simulink model "sma_corr"  (Simulink 8.0)
settings: Solver=VariableStepDiscrete  SolverMode=SingleTasking  StartTime=0.0  StopTime=1000  RelTol=1e-3
PreLoadFcn:
  | % Set this to 1 if simulating
  | simulating = 0;
  | 
  | % Overall parameters
  | n_comp = 128;
  | 
  | % Simulation
  | if (simulating)
  |     n_tvg_frames = 4;
  |     frames_per_vector = 4*8;
  |     vectors_per_wstep = 2;
  |     total_wsteps = 1; % 2^n
  |     % Laura's params
  |     x_ctr = 12;
  |     x_data_ctr = x_ctr-5;
  |     rx_addr_ctr = x_data_ctr+2;
  |     tx_addr_ctr = x_data_ctr+2;
  | else
  |     n_tvg_frames = 256;
  |     frames_per_vector = 256*8;
  |     vectors_per_wstep = 11;
  |     total_wsteps = 6; % 2^n
  |     % Laura's params
  |     x_ctr = 32;
  |     x_data_ctr = 18;
  |     rx_addr_ctr = x_data_ctr+2;
  |     tx_addr_ctr = x_data_ctr+2;
  | end
  | 
  | sb_states = repmat([ones(1, 256) zeros(1, 256)], 1, 2^(total_wsteps-1));
InitFcn:
  | % Get the XSG object to find the clock source
  | xps_xsg_blks = find_system(gcs,'FollowLinks','on','LookUnderMasks','all', 'SearchDepth',1, 'Tag','xps:xsg');
  | xsg_obj = xps_block(xps_xsg_blks{1}, {});
  | xsg_obj = xps_xsg(xsg_obj);
  | clk_src = get(xsg_obj, 'clk_src');
  | if strcmp(clk_src, 'adc0_clk')
  |     adc_brd = 'ZDOK 0';
  | elseif strcmp(clk_src, 'adc1_clk')
  |     adc_brd = 'ZDOK 1';
  | else
  |     adc_brd = '';
  | end
  | 
  | adc_clk_rate = 0;
  | % Get the appropriate ADC5g object
  | xps_adc5g_blks = find_system(gcs, 'FollowLinks', 'on', 'LookUnderMasks','all', 'SearchDepth',2, 'Tag','xps:adc5g');
  | for n = 1:length(xps_adc5g_blks)
  |     adc5g_blk = xps_adc5g_blks{n};
  |     if strcmp(get_param(adc5g_blk, 'adc_brd'), adc_brd)
  |         adc_clk_rate = 2*str2num(get_param(adc5g_blk, 'adc_clk_rate'));
  |     end
  | end
undefined symbols (not defined in the file or in its folder): Down, sfp, Up, X, xlipgetpartsetting, Z
libraries: xbsIndex_r4 x9231, casper_library_misc x178, dspsigops x32, casper_library_delays x26, xps_library x21
(part 52 of 92)

== system sma_corr/beng/sum2/sum_Xcd/gain_and_quantize ==
  note: w,x,y,z are Fix_8_0, but the MSb is redundant
      | (they are really only Fix_7_0).  We want to keep
      | the top 4 of those seven bits, so we reinterpret
      | them as Fix_8_3 before applying a UFix_8_4 gain
      | which gives Fix_16_7.  The Fix_16_7 value is cast
      | to a Fix_4_0 with saturation and round-to-even.
  g : Inport
  in8 : Inport  Port=2
  Mult : xbsIndex_r4/Mult  precision=Full  arith_type="Signed  (2's comp)"  n_bits=16  bin_pt=14  quantization=Truncate  overflow=Wrap  en=off  latency=3  dbl_ovrd=off  use_behavioral_HDL=off  opt=Speed  use_embedded=on  optimum_pipeline=off  pipeline=on  use_rpm=on  placement_style=Triangular  block_type=mult
  Reinterpret1 : xbsIndex_r4/Reinterpret  force_arith_type=off  arith_type=Unsigned  force_bin_pt=on  bin_pt=3  block_type=reinterpret
  convert : Subsystem  AncestorBlock=casper_library_misc/convert  mask(convert){bin_pt_in=7, n_bits_out=4, bin_pt_out=0, quantization=Round  (unbiased: Even Values), overflow=Saturate, latency=4}
      mask prompts: bin_pt_in "Binary Point In"; n_bits_out "Bit Width Out"; bin_pt_out "Binary Point Out"
      mask help:
      | Convert input value to the specified bit width and precision.
      |
      | Does not support upscaling number of fractional bits (eg 8_5 to 8_4 is ok, but not 8_4 to 8_5).
      mask init: same as sma_corr/cgain_0/convert
      settings: AttributesFormatString=|
      | N_7 ==> 4_0
      | round even, saturate
      | latency = 4
      mask Help:
      | <pre>
      | Description::
      | 
      | Outputs converted version of the input.
      | 
      | Mask Parameters::
      | 
      | Binary Point In (bin_pt_in): Specifies the binary point location in the input.
      | 
      | Bit Width Out (n_bits_out): Specifies the width of the output.
      | 
      | Binary Point Out (bin_pt_out): Specifies the binary point location in the output.
      | 
      | Quantization (quantization): Specifies quantization handling.
      | 
      | Overflow (overflow): Specifies overflow handling.
      | 
      | Latency (latency): Specifies the latency of the underlying adder block, which is the overall
      | latency of this block as well.
      | </pre>
      mask TypeOptions quantization: Truncate | Round  (unbiased: +/- Inf) | Round  (unbiased: Even Values) | Round  (unbiased: Zero) | Round  (unbiased: Odd Values) | Round  (biased: Up) | Round  (biased: Down)
      mask TypeOptions overflow: Wrap | Saturate | Error
  out4 : Outport
  wires:
  g/1 -> Mult/1
  in8/1 -> Reinterpret1/1
  Mult/1 -> convert/1(in)
  Reinterpret1/1 -> Mult/2
  convert/1(out) -> out4/1

== system sma_corr/beng/sum2/sum_Xcd/gain_and_quantize1 ==
  note: w,x,y,z are Fix_8_0, but the MSb is redundant
      | (they are really only Fix_7_0).  We want to keep
      | the top 4 of those seven bits, so we reinterpret
      | them as Fix_8_3 before applying a UFix_8_4 gain
      | which gives Fix_16_7.  The Fix_16_7 value is cast
      | to a Fix_4_0 with saturation and round-to-even.
  g : Inport
  in8 : Inport  Port=2
  Mult : xbsIndex_r4/Mult  precision=Full  arith_type="Signed  (2's comp)"  n_bits=16  bin_pt=14  quantization=Truncate  overflow=Wrap  en=off  latency=3  dbl_ovrd=off  use_behavioral_HDL=off  opt=Speed  use_embedded=on  optimum_pipeline=off  pipeline=on  use_rpm=on  placement_style=Triangular  block_type=mult
  Reinterpret1 : xbsIndex_r4/Reinterpret  force_arith_type=off  arith_type=Unsigned  force_bin_pt=on  bin_pt=3  block_type=reinterpret
  convert : Subsystem  AncestorBlock=casper_library_misc/convert  mask(convert){bin_pt_in=7, n_bits_out=4, bin_pt_out=0, quantization=Round  (unbiased: Even Values), overflow=Saturate, latency=4}
      mask prompts: bin_pt_in "Binary Point In"; n_bits_out "Bit Width Out"; bin_pt_out "Binary Point Out"
      mask help:
      | Convert input value to the specified bit width and precision.
      |
      | Does not support upscaling number of fractional bits (eg 8_5 to 8_4 is ok, but not 8_4 to 8_5).
      mask init: same as sma_corr/cgain_0/convert
      settings: AttributesFormatString=|
      | N_7 ==> 4_0
      | round even, saturate
      | latency = 4
      mask Help:
      | <pre>
      | Description::
      | 
      | Outputs converted version of the input.
      | 
      | Mask Parameters::
      | 
      | Binary Point In (bin_pt_in): Specifies the binary point location in the input.
      | 
      | Bit Width Out (n_bits_out): Specifies the width of the output.
      | 
      | Binary Point Out (bin_pt_out): Specifies the binary point location in the output.
      | 
      | Quantization (quantization): Specifies quantization handling.
      | 
      | Overflow (overflow): Specifies overflow handling.
      | 
      | Latency (latency): Specifies the latency of the underlying adder block, which is the overall
      | latency of this block as well.
      | </pre>
      mask TypeOptions quantization: Truncate | Round  (unbiased: +/- Inf) | Round  (unbiased: Even Values) | Round  (unbiased: Zero) | Round  (unbiased: Odd Values) | Round  (biased: Up) | Round  (biased: Down)
      mask TypeOptions overflow: Wrap | Saturate | Error
  out4 : Outport
  wires:
  convert/1(out) -> out4/1
  Reinterpret1/1 -> Mult/2
  Mult/1 -> convert/1(in)
  in8/1 -> Reinterpret1/1
  g/1 -> Mult/1

== system sma_corr/beng/sum2/sum_Xcd/gain_and_quantize2 ==
  note: w,x,y,z are Fix_8_0, but the MSb is redundant
      | (they are really only Fix_7_0).  We want to keep
      | the top 4 of those seven bits, so we reinterpret
      | them as Fix_8_3 before applying a UFix_8_4 gain
      | which gives Fix_16_7.  The Fix_16_7 value is cast
      | to a Fix_4_0 with saturation and round-to-even.
  g : Inport
  in8 : Inport  Port=2
  Mult : xbsIndex_r4/Mult  precision=Full  arith_type="Signed  (2's comp)"  n_bits=16  bin_pt=14  quantization=Truncate  overflow=Wrap  en=off  latency=3  dbl_ovrd=off  use_behavioral_HDL=off  opt=Speed  use_embedded=on  optimum_pipeline=off  pipeline=on  use_rpm=on  placement_style=Triangular  block_type=mult
  Reinterpret1 : xbsIndex_r4/Reinterpret  force_arith_type=off  arith_type=Unsigned  force_bin_pt=on  bin_pt=3  block_type=reinterpret
  convert : Subsystem  AncestorBlock=casper_library_misc/convert  mask(convert){bin_pt_in=7, n_bits_out=4, bin_pt_out=0, quantization=Round  (unbiased: Even Values), overflow=Saturate, latency=4}
      mask prompts: bin_pt_in "Binary Point In"; n_bits_out "Bit Width Out"; bin_pt_out "Binary Point Out"
      mask help:
      | Convert input value to the specified bit width and precision.
      |
      | Does not support upscaling number of fractional bits (eg 8_5 to 8_4 is ok, but not 8_4 to 8_5).
      mask init: same as sma_corr/cgain_0/convert
      settings: AttributesFormatString=|
      | N_7 ==> 4_0
      | round even, saturate
      | latency = 4
      mask Help:
      | <pre>
      | Description::
      | 
      | Outputs converted version of the input.
      | 
      | Mask Parameters::
      | 
      | Binary Point In (bin_pt_in): Specifies the binary point location in the input.
      | 
      | Bit Width Out (n_bits_out): Specifies the width of the output.
      | 
      | Binary Point Out (bin_pt_out): Specifies the binary point location in the output.
      | 
      | Quantization (quantization): Specifies quantization handling.
      | 
      | Overflow (overflow): Specifies overflow handling.
      | 
      | Latency (latency): Specifies the latency of the underlying adder block, which is the overall
      | latency of this block as well.
      | </pre>
      mask TypeOptions quantization: Truncate | Round  (unbiased: +/- Inf) | Round  (unbiased: Even Values) | Round  (unbiased: Zero) | Round  (unbiased: Odd Values) | Round  (biased: Up) | Round  (biased: Down)
      mask TypeOptions overflow: Wrap | Saturate | Error
  out4 : Outport
  wires:
  g/1 -> Mult/1
  in8/1 -> Reinterpret1/1
  Mult/1 -> convert/1(in)
  Reinterpret1/1 -> Mult/2
  convert/1(out) -> out4/1

== system sma_corr/beng/sum2/sum_Xcd/gain_and_quantize3 ==
  note: w,x,y,z are Fix_8_0, but the MSb is redundant
      | (they are really only Fix_7_0).  We want to keep
      | the top 4 of those seven bits, so we reinterpret
      | them as Fix_8_3 before applying a UFix_8_4 gain
      | which gives Fix_16_7.  The Fix_16_7 value is cast
      | to a Fix_4_0 with saturation and round-to-even.
  g : Inport
  in8 : Inport  Port=2
  Mult : xbsIndex_r4/Mult  precision=Full  arith_type="Signed  (2's comp)"  n_bits=16  bin_pt=14  quantization=Truncate  overflow=Wrap  en=off  latency=3  dbl_ovrd=off  use_behavioral_HDL=off  opt=Speed  use_embedded=on  optimum_pipeline=off  pipeline=on  use_rpm=on  placement_style=Triangular  block_type=mult
  Reinterpret1 : xbsIndex_r4/Reinterpret  force_arith_type=off  arith_type=Unsigned  force_bin_pt=on  bin_pt=3  block_type=reinterpret
  convert : Subsystem  AncestorBlock=casper_library_misc/convert  mask(convert){bin_pt_in=7, n_bits_out=4, bin_pt_out=0, quantization=Round  (unbiased: Even Values), overflow=Saturate, latency=4}
      mask prompts: bin_pt_in "Binary Point In"; n_bits_out "Bit Width Out"; bin_pt_out "Binary Point Out"
      mask help:
      | Convert input value to the specified bit width and precision.
      |
      | Does not support upscaling number of fractional bits (eg 8_5 to 8_4 is ok, but not 8_4 to 8_5).
      mask init: same as sma_corr/cgain_0/convert
      settings: AttributesFormatString=|
      | N_7 ==> 4_0
      | round even, saturate
      | latency = 4
      mask Help:
      | <pre>
      | Description::
      | 
      | Outputs converted version of the input.
      | 
      | Mask Parameters::
      | 
      | Binary Point In (bin_pt_in): Specifies the binary point location in the input.
      | 
      | Bit Width Out (n_bits_out): Specifies the width of the output.
      | 
      | Binary Point Out (bin_pt_out): Specifies the binary point location in the output.
      | 
      | Quantization (quantization): Specifies quantization handling.
      | 
      | Overflow (overflow): Specifies overflow handling.
      | 
      | Latency (latency): Specifies the latency of the underlying adder block, which is the overall
      | latency of this block as well.
      | </pre>
      mask TypeOptions quantization: Truncate | Round  (unbiased: +/- Inf) | Round  (unbiased: Even Values) | Round  (unbiased: Zero) | Round  (unbiased: Odd Values) | Round  (biased: Up) | Round  (biased: Down)
      mask TypeOptions overflow: Wrap | Saturate | Error
  out4 : Outport
  wires:
  convert/1(out) -> out4/1
  Reinterpret1/1 -> Mult/2
  Mult/1 -> convert/1(in)
  in8/1 -> Reinterpret1/1
  g/1 -> Mult/1

== system sma_corr/beng/sum2/sum_Xcd/simd_vacc ==
  note: Note that this bit basher takes the upper 8 bits of every 12, as opposed to the
      | lower 8, which is the case in .../sum[01]/sum_???/simd_vac (sideband0 path).
      | Here the upper 8 are needed, since we accumulate 8-bit data over 8 cycles -> grows to 12-bit;
      | there the lower 8 are needed, since it accumulates 4-bit data over 8 cycles -> grows to 8-bit.
  note: First "acc_init" pulse after sync does not indicate valid.
      | Hold off "valid" output until after the first falling edge of
      | acc_init that is after the sync pulse.
  note: Accummulate 8 vectors of length 128 samples.
      | Do four such accumulations in parallel.
  sync : Inport
  in : Inport  Port=2
  disable : Inport  Port=3
  BitBasher2 : xbsIndex_r4/BitBasher  bitexpr=|  display_expr=off  sr_1=1  arith_type1="Signed  (2's comp)"  bin_pt1=0  sr_2=2  arith_type2="Signed  (2's comp)"  bin_pt2=0  sr_3=3  arith_type3="Signed  (2's comp)"  bin_pt3=0  sr_4=4  arith_type4="Signed  (2's comp)"  bin_pt4=0  dbl_ovrd=off  block_type=bitbasher
      | <!DOCTYPE HTML PUBLIC "-//W3C//DTD HTML 4.0//EN" "http://www.w3.org/TR/REC-html40/strict.dtd">
      | <html><head><meta name="qrichtext" content="1" /><style type="text/css">
      | p, li { white-space: pre-wrap; }
      | </style></head><body style=" font-family:'Ubuntu'; font-size:11pt; font-weight:400; font-style:normal;">
      | <p style=" margin-top:12px; margin-bottom:12px; margin-left:0px; margin-right:0px; -qt-block-indent:0; text-indent:0px;"><span style=" font-family:'MS Shell Dlg'; font-size:8pt;">w={p[47:40]}<br />x={p[35:28]}<br />y={p[23:16]}<br />z={p[11:04]}<br /></span></p></body></html>
  Constant1 : xbsIndex_r4/Constant  const=1  gui_display_data_type=Boolean  arith_type=Boolean  n_bits=16  bin_pt=14  preci_type=Single  exp_width=8  frac_width=24  explicit_period=on  period=1  equ=P=C  opselect=C  inp2=PCIN>>17  opr=+  inp1=P  carry=CIN  dbl_ovrd=off  block_type=constant
  Delay{1..4} (4 alike) : xbsIndex_r4/Delay  rst=off  infoeditControl=Selection of Reset will increase slice count due to use of real FFs and instead of SRLs  en=off  latency=1  dbl_ovrd=off  reg_retiming=off  block_type=delay
  Register1 : xbsIndex_r4/Register  init=0  rst=on  en=on  dbl_ovrd=off  block_type=register
  acc4par : Subsystem
  armed_trigger : casper_library_misc/armed_trigger
  controller : Subsystem
  delay_bram : Subsystem  AncestorBlock=casper_library_delays/delay_bram  mask(delay_bram){DelayLen=128-4, bram_latency=3, use_dsp48=off, async=off}
      mask prompts: DelayLen "Delay By:"; bram_latency "BRAM Latency"; use_dsp48 "Use DSP48"; async "asynchronous operation"
      mask help: A delay block that uses BRAM for its storage.
      mask init:
      | delay_bram_init(gcb, ...
      |     'DelayLen', DelayLen, ...
      |     'bram_latency', bram_latency, ...
      |     'use_dsp48', use_dsp48, ...
      |     'async', async);
      mask Help: eval('xlWeb(''http://casper.berkeley.edu/wiki/Delay_bram'')')
  edge_detect : Subsystem  AncestorBlock=casper_library_misc/edge_detect  mask(edge_detect){edge=Falling, polarity=Active High, x_in=[0 0.08 0.08 0.16 0.16 0.24 0.24 0.32 0.32 0.4], y_in=[-1 -1  1  1  1  1  1  1  1  1], x_out=[0.6 0.68 0.68 0.76 0.76 0.84 0.84 0.92 0.92 1], y_out=[-1 -1  1  1 -1 -1 -1 -1 -1 -1]}
      mask prompts: edge "Edge Type"; polarity "Output Polarity"; x_in "Input X Positions"; y_in "Input Y Positions"; x_out "Output X Positions"; y_out "Output Y Positions"
      mask help: Output is active for one-cycle after each edge of the specified type (i.e. rising, falling or both).
      mask init: same as sma_corr/scope/edge_detect
      settings: AttributesFormatString=|
      | falling edges
      | active high
      mask Help: Output is active for one-cycle after each edge of the specified type (i.e. rising, falling, or both).  Latency is zero.
      mask TypeOptions edge: Rising | Falling | Both
      mask TypeOptions polarity: Active High | Active Low
      mask Display:
      | plot(x_in_num,0.5+y_in_num*0.2);
      | plot(x_out_num,0.5+y_out_num*0.2);
  valid_holdoff : xbsIndex_r4/Logical  logical_function=AND  inputs=2  en=off  latency=1  precision=Full  arith_type=Unsigned  n_bits=16  bin_pt=0  align_bp=on  dbl_ovrd=off  block_type=logical
  sync_out : Outport
  valid : Outport  Port=2
  w : Outport  Port=3
  x : Outport  Port=4
  y : Outport  Port=5
  z : Outport  Port=6
  wires:
  in/1 -> Delay3/1
  delay_bram/1(dout) -> Delay2/1
  acc4par/1(out48) -> Delay1/1
  Delay1/1 -> delay_bram/1(din)
  Delay2/1 -> acc4par/4(acc48), Delay4/1
  Delay4/1 -> BitBasher2/1
  edge_detect/1(out) -> Register1/3
  Constant1/1 -> Register1/1
  Register1/1 -> armed_trigger/1, valid_holdoff/2
  controller/2(acc_init) -> edge_detect/1(in), acc4par/3(acc_init), valid_holdoff/1, armed_trigger/2
  sync/1 -> Register1/2, controller/2(sync)
  disable/1 -> controller/1(disable)
  controller/1(zero_op) -> acc4par/2(zero_input)
  Delay3/1 -> acc4par/1(in32)
  valid_holdoff/1 -> valid/1
  armed_trigger/1 -> sync_out/1
  BitBasher2/1 -> w/1
  BitBasher2/4 -> z/1
  BitBasher2/2 -> x/1
  BitBasher2/3 -> y/1

== system sma_corr/beng/sum2/sum_Yab/gain_and_quantize ==
  note: w,x,y,z are Fix_8_0, but the MSb is redundant
      | (they are really only Fix_7_0).  We want to keep
      | the top 4 of those seven bits, so we reinterpret
      | them as Fix_8_3 before applying a UFix_8_4 gain
      | which gives Fix_16_7.  The Fix_16_7 value is cast
      | to a Fix_4_0 with saturation and round-to-even.
  g : Inport
  in8 : Inport  Port=2
  Mult : xbsIndex_r4/Mult  precision=Full  arith_type="Signed  (2's comp)"  n_bits=16  bin_pt=14  quantization=Truncate  overflow=Wrap  en=off  latency=3  dbl_ovrd=off  use_behavioral_HDL=off  opt=Speed  use_embedded=on  optimum_pipeline=off  pipeline=on  use_rpm=on  placement_style=Triangular  block_type=mult
  Reinterpret1 : xbsIndex_r4/Reinterpret  force_arith_type=off  arith_type=Unsigned  force_bin_pt=on  bin_pt=3  block_type=reinterpret
  convert : Subsystem  AncestorBlock=casper_library_misc/convert  mask(convert){bin_pt_in=7, n_bits_out=4, bin_pt_out=0, quantization=Round  (unbiased: Even Values), overflow=Saturate, latency=4}
      mask prompts: bin_pt_in "Binary Point In"; n_bits_out "Bit Width Out"; bin_pt_out "Binary Point Out"
      mask help:
      | Convert input value to the specified bit width and precision.
      |
      | Does not support upscaling number of fractional bits (eg 8_5 to 8_4 is ok, but not 8_4 to 8_5).
      mask init: same as sma_corr/cgain_0/convert
      settings: AttributesFormatString=|
      | N_7 ==> 4_0
      | round even, saturate
      | latency = 4
      mask Help:
      | <pre>
      | Description::
      | 
      | Outputs converted version of the input.
      | 
      | Mask Parameters::
      | 
      | Binary Point In (bin_pt_in): Specifies the binary point location in the input.
      | 
      | Bit Width Out (n_bits_out): Specifies the width of the output.
      | 
      | Binary Point Out (bin_pt_out): Specifies the binary point location in the output.
      | 
      | Quantization (quantization): Specifies quantization handling.
      | 
      | Overflow (overflow): Specifies overflow handling.
      | 
      | Latency (latency): Specifies the latency of the underlying adder block, which is the overall
      | latency of this block as well.
      | </pre>
      mask TypeOptions quantization: Truncate | Round  (unbiased: +/- Inf) | Round  (unbiased: Even Values) | Round  (unbiased: Zero) | Round  (unbiased: Odd Values) | Round  (biased: Up) | Round  (biased: Down)
      mask TypeOptions overflow: Wrap | Saturate | Error
  out4 : Outport
  wires:
  g/1 -> Mult/1
  in8/1 -> Reinterpret1/1
  Mult/1 -> convert/1(in)
  Reinterpret1/1 -> Mult/2
  convert/1(out) -> out4/1

== system sma_corr/beng/sum2/sum_Yab/gain_and_quantize1 ==
  note: w,x,y,z are Fix_8_0, but the MSb is redundant
      | (they are really only Fix_7_0).  We want to keep
      | the top 4 of those seven bits, so we reinterpret
      | them as Fix_8_3 before applying a UFix_8_4 gain
      | which gives Fix_16_7.  The Fix_16_7 value is cast
      | to a Fix_4_0 with saturation and round-to-even.
  g : Inport
  in8 : Inport  Port=2
  Mult : xbsIndex_r4/Mult  precision=Full  arith_type="Signed  (2's comp)"  n_bits=16  bin_pt=14  quantization=Truncate  overflow=Wrap  en=off  latency=3  dbl_ovrd=off  use_behavioral_HDL=off  opt=Speed  use_embedded=on  optimum_pipeline=off  pipeline=on  use_rpm=on  placement_style=Triangular  block_type=mult
  Reinterpret1 : xbsIndex_r4/Reinterpret  force_arith_type=off  arith_type=Unsigned  force_bin_pt=on  bin_pt=3  block_type=reinterpret
  convert : Subsystem  AncestorBlock=casper_library_misc/convert  mask(convert){bin_pt_in=7, n_bits_out=4, bin_pt_out=0, quantization=Round  (unbiased: Even Values), overflow=Saturate, latency=4}
      mask prompts: bin_pt_in "Binary Point In"; n_bits_out "Bit Width Out"; bin_pt_out "Binary Point Out"
      mask help:
      | Convert input value to the specified bit width and precision.
      |
      | Does not support upscaling number of fractional bits (eg 8_5 to 8_4 is ok, but not 8_4 to 8_5).
      mask init: same as sma_corr/cgain_0/convert
      settings: AttributesFormatString=|
      | N_7 ==> 4_0
      | round even, saturate
      | latency = 4
      mask Help:
      | <pre>
      | Description::
      | 
      | Outputs converted version of the input.
      | 
      | Mask Parameters::
      | 
      | Binary Point In (bin_pt_in): Specifies the binary point location in the input.
      | 
      | Bit Width Out (n_bits_out): Specifies the width of the output.
      | 
      | Binary Point Out (bin_pt_out): Specifies the binary point location in the output.
      | 
      | Quantization (quantization): Specifies quantization handling.
      | 
      | Overflow (overflow): Specifies overflow handling.
      | 
      | Latency (latency): Specifies the latency of the underlying adder block, which is the overall
      | latency of this block as well.
      | </pre>
      mask TypeOptions quantization: Truncate | Round  (unbiased: +/- Inf) | Round  (unbiased: Even Values) | Round  (unbiased: Zero) | Round  (unbiased: Odd Values) | Round  (biased: Up) | Round  (biased: Down)
      mask TypeOptions overflow: Wrap | Saturate | Error
  out4 : Outport
  wires:
  convert/1(out) -> out4/1
  Reinterpret1/1 -> Mult/2
  Mult/1 -> convert/1(in)
  in8/1 -> Reinterpret1/1
  g/1 -> Mult/1

== system sma_corr/beng/sum2/sum_Yab/gain_and_quantize2 ==
  note: w,x,y,z are Fix_8_0, but the MSb is redundant
      | (they are really only Fix_7_0).  We want to keep
      | the top 4 of those seven bits, so we reinterpret
      | them as Fix_8_3 before applying a UFix_8_4 gain
      | which gives Fix_16_7.  The Fix_16_7 value is cast
      | to a Fix_4_0 with saturation and round-to-even.
  g : Inport
  in8 : Inport  Port=2
  Mult : xbsIndex_r4/Mult  precision=Full  arith_type="Signed  (2's comp)"  n_bits=16  bin_pt=14  quantization=Truncate  overflow=Wrap  en=off  latency=3  dbl_ovrd=off  use_behavioral_HDL=off  opt=Speed  use_embedded=on  optimum_pipeline=off  pipeline=on  use_rpm=on  placement_style=Triangular  block_type=mult
  Reinterpret1 : xbsIndex_r4/Reinterpret  force_arith_type=off  arith_type=Unsigned  force_bin_pt=on  bin_pt=3  block_type=reinterpret
  convert : Subsystem  AncestorBlock=casper_library_misc/convert  mask(convert){bin_pt_in=7, n_bits_out=4, bin_pt_out=0, quantization=Round  (unbiased: Even Values), overflow=Saturate, latency=4}
      mask prompts: bin_pt_in "Binary Point In"; n_bits_out "Bit Width Out"; bin_pt_out "Binary Point Out"
      mask help:
      | Convert input value to the specified bit width and precision.
      |
      | Does not support upscaling number of fractional bits (eg 8_5 to 8_4 is ok, but not 8_4 to 8_5).
      mask init: same as sma_corr/cgain_0/convert
      settings: AttributesFormatString=|
      | N_7 ==> 4_0
      | round even, saturate
      | latency = 4
      mask Help:
      | <pre>
      | Description::
      | 
      | Outputs converted version of the input.
      | 
      | Mask Parameters::
      | 
      | Binary Point In (bin_pt_in): Specifies the binary point location in the input.
      | 
      | Bit Width Out (n_bits_out): Specifies the width of the output.
      | 
      | Binary Point Out (bin_pt_out): Specifies the binary point location in the output.
      | 
      | Quantization (quantization): Specifies quantization handling.
      | 
      | Overflow (overflow): Specifies overflow handling.
      | 
      | Latency (latency): Specifies the latency of the underlying adder block, which is the overall
      | latency of this block as well.
      | </pre>
      mask TypeOptions quantization: Truncate | Round  (unbiased: +/- Inf) | Round  (unbiased: Even Values) | Round  (unbiased: Zero) | Round  (unbiased: Odd Values) | Round  (biased: Up) | Round  (biased: Down)
      mask TypeOptions overflow: Wrap | Saturate | Error
  out4 : Outport
  wires:
  g/1 -> Mult/1
  in8/1 -> Reinterpret1/1
  Mult/1 -> convert/1(in)
  Reinterpret1/1 -> Mult/2
  convert/1(out) -> out4/1

== system sma_corr/beng/sum2/sum_Yab/gain_and_quantize3 ==
  note: w,x,y,z are Fix_8_0, but the MSb is redundant
      | (they are really only Fix_7_0).  We want to keep
      | the top 4 of those seven bits, so we reinterpret
      | them as Fix_8_3 before applying a UFix_8_4 gain
      | which gives Fix_16_7.  The Fix_16_7 value is cast
      | to a Fix_4_0 with saturation and round-to-even.
  g : Inport
  in8 : Inport  Port=2
  Mult : xbsIndex_r4/Mult  precision=Full  arith_type="Signed  (2's comp)"  n_bits=16  bin_pt=14  quantization=Truncate  overflow=Wrap  en=off  latency=3  dbl_ovrd=off  use_behavioral_HDL=off  opt=Speed  use_embedded=on  optimum_pipeline=off  pipeline=on  use_rpm=on  placement_style=Triangular  block_type=mult
  Reinterpret1 : xbsIndex_r4/Reinterpret  force_arith_type=off  arith_type=Unsigned  force_bin_pt=on  bin_pt=3  block_type=reinterpret
  convert : Subsystem  AncestorBlock=casper_library_misc/convert  mask(convert){bin_pt_in=7, n_bits_out=4, bin_pt_out=0, quantization=Round  (unbiased: Even Values), overflow=Saturate, latency=4}
      mask prompts: bin_pt_in "Binary Point In"; n_bits_out "Bit Width Out"; bin_pt_out "Binary Point Out"
      mask help:
      | Convert input value to the specified bit width and precision.
      |
      | Does not support upscaling number of fractional bits (eg 8_5 to 8_4 is ok, but not 8_4 to 8_5).
      mask init: same as sma_corr/cgain_0/convert
      settings: AttributesFormatString=|
      | N_7 ==> 4_0
      | round even, saturate
      | latency = 4
      mask Help:
      | <pre>
      | Description::
      | 
      | Outputs converted version of the input.
      | 
      | Mask Parameters::
      | 
      | Binary Point In (bin_pt_in): Specifies the binary point location in the input.
      | 
      | Bit Width Out (n_bits_out): Specifies the width of the output.
      | 
      | Binary Point Out (bin_pt_out): Specifies the binary point location in the output.
      | 
      | Quantization (quantization): Specifies quantization handling.
      | 
      | Overflow (overflow): Specifies overflow handling.
      | 
      | Latency (latency): Specifies the latency of the underlying adder block, which is the overall
      | latency of this block as well.
      | </pre>
      mask TypeOptions quantization: Truncate | Round  (unbiased: +/- Inf) | Round  (unbiased: Even Values) | Round  (unbiased: Zero) | Round  (unbiased: Odd Values) | Round  (biased: Up) | Round  (biased: Down)
      mask TypeOptions overflow: Wrap | Saturate | Error
  out4 : Outport
  wires:
  convert/1(out) -> out4/1
  Reinterpret1/1 -> Mult/2
  Mult/1 -> convert/1(in)
  in8/1 -> Reinterpret1/1
  g/1 -> Mult/1

== system sma_corr/beng/sum2/sum_Yab/simd_vacc ==
  note: Note that this bit basher takes the upper 8 bits of every 12, as opposed to the
      | lower 8, which is the case in .../sum[01]/sum_???/simd_vac (sideband0 path).
      | Here the upper 8 are needed, since we accumulate 8-bit data over 8 cycles -> grows to 12-bit;
      | there the lower 8 are needed, since it accumulates 4-bit data over 8 cycles -> grows to 8-bit.
  note: First "acc_init" pulse after sync does not indicate valid.
      | Hold off "valid" output until after the first falling edge of
      | acc_init that is after the sync pulse.
  note: Accummulate 8 vectors of length 128 samples.
      | Do four such accumulations in parallel.
  sync : Inport
  in : Inport  Port=2
  disable : Inport  Port=3
  BitBasher2 : xbsIndex_r4/BitBasher  bitexpr=|  display_expr=off  sr_1=1  arith_type1="Signed  (2's comp)"  bin_pt1=0  sr_2=2  arith_type2="Signed  (2's comp)"  bin_pt2=0  sr_3=3  arith_type3="Signed  (2's comp)"  bin_pt3=0  sr_4=4  arith_type4="Signed  (2's comp)"  bin_pt4=0  dbl_ovrd=off  block_type=bitbasher
      | <!DOCTYPE HTML PUBLIC "-//W3C//DTD HTML 4.0//EN" "http://www.w3.org/TR/REC-html40/strict.dtd">
      | <html><head><meta name="qrichtext" content="1" /><style type="text/css">
      | p, li { white-space: pre-wrap; }
      | </style></head><body style=" font-family:'Ubuntu'; font-size:11pt; font-weight:400; font-style:normal;">
      | <p style=" margin-top:12px; margin-bottom:12px; margin-left:0px; margin-right:0px; -qt-block-indent:0; text-indent:0px;"><span style=" font-family:'MS Shell Dlg'; font-size:8pt;">w={p[47:40]}<br />x={p[35:28]}<br />y={p[23:16]}<br />z={p[11:04]}<br /></span></p></body></html>
  Constant1 : xbsIndex_r4/Constant  const=1  gui_display_data_type=Boolean  arith_type=Boolean  n_bits=16  bin_pt=14  preci_type=Single  exp_width=8  frac_width=24  explicit_period=on  period=1  equ=P=C  opselect=C  inp2=PCIN>>17  opr=+  inp1=P  carry=CIN  dbl_ovrd=off  block_type=constant
  Delay{1..4} (4 alike) : xbsIndex_r4/Delay  rst=off  infoeditControl=Selection of Reset will increase slice count due to use of real FFs and instead of SRLs  en=off  latency=1  dbl_ovrd=off  reg_retiming=off  block_type=delay
  Register1 : xbsIndex_r4/Register  init=0  rst=on  en=on  dbl_ovrd=off  block_type=register
  acc4par : Subsystem
  armed_trigger : casper_library_misc/armed_trigger
  controller : Subsystem
  delay_bram : Subsystem  AncestorBlock=casper_library_delays/delay_bram  mask(delay_bram){DelayLen=128-4, bram_latency=3, use_dsp48=off, async=off}
      mask prompts: DelayLen "Delay By:"; bram_latency "BRAM Latency"; use_dsp48 "Use DSP48"; async "asynchronous operation"
      mask help: A delay block that uses BRAM for its storage.
      mask init:
      | delay_bram_init(gcb, ...
      |     'DelayLen', DelayLen, ...
      |     'bram_latency', bram_latency, ...
      |     'use_dsp48', use_dsp48, ...
      |     'async', async);
      mask Help: eval('xlWeb(''http://casper.berkeley.edu/wiki/Delay_bram'')')
  edge_detect : Subsystem  AncestorBlock=casper_library_misc/edge_detect  mask(edge_detect){edge=Falling, polarity=Active High, x_in=[0 0.08 0.08 0.16 0.16 0.24 0.24 0.32 0.32 0.4], y_in=[-1 -1  1  1  1  1  1  1  1  1], x_out=[0.6 0.68 0.68 0.76 0.76 0.84 0.84 0.92 0.92 1], y_out=[-1 -1  1  1 -1 -1 -1 -1 -1 -1]}
      mask prompts: edge "Edge Type"; polarity "Output Polarity"; x_in "Input X Positions"; y_in "Input Y Positions"; x_out "Output X Positions"; y_out "Output Y Positions"
      mask help: Output is active for one-cycle after each edge of the specified type (i.e. rising, falling or both).
      mask init: same as sma_corr/scope/edge_detect
      settings: AttributesFormatString=|
      | falling edges
      | active high
      mask Help: Output is active for one-cycle after each edge of the specified type (i.e. rising, falling, or both).  Latency is zero.
      mask TypeOptions edge: Rising | Falling | Both
      mask TypeOptions polarity: Active High | Active Low
      mask Display:
      | plot(x_in_num,0.5+y_in_num*0.2);
      | plot(x_out_num,0.5+y_out_num*0.2);
  valid_holdoff : xbsIndex_r4/Logical  logical_function=AND  inputs=2  en=off  latency=1  precision=Full  arith_type=Unsigned  n_bits=16  bin_pt=0  align_bp=on  dbl_ovrd=off  block_type=logical
  sync_out : Outport
  valid : Outport  Port=2
  w : Outport  Port=3
  x : Outport  Port=4
  y : Outport  Port=5
  z : Outport  Port=6
  wires:
  in/1 -> Delay3/1
  delay_bram/1(dout) -> Delay2/1
  acc4par/1(out48) -> Delay1/1
  Delay1/1 -> delay_bram/1(din)
  Delay2/1 -> acc4par/4(acc48), Delay4/1
  Delay4/1 -> BitBasher2/1
  edge_detect/1(out) -> Register1/3
  Constant1/1 -> Register1/1
  Register1/1 -> armed_trigger/1, valid_holdoff/2
  controller/2(acc_init) -> edge_detect/1(in), acc4par/3(acc_init), valid_holdoff/1, armed_trigger/2
  sync/1 -> Register1/2, controller/2(sync)
  disable/1 -> controller/1(disable)
  controller/1(zero_op) -> acc4par/2(zero_input)
  Delay3/1 -> acc4par/1(in32)
  valid_holdoff/1 -> valid/1
  armed_trigger/1 -> sync_out/1
  BitBasher2/1 -> w/1
  BitBasher2/4 -> z/1
  BitBasher2/2 -> x/1
  BitBasher2/3 -> y/1

== system sma_corr/beng/sum2/sum_Ycd/gain_and_quantize ==
  note: w,x,y,z are Fix_8_0, but the MSb is redundant
      | (they are really only Fix_7_0).  We want to keep
      | the top 4 of those seven bits, so we reinterpret
      | them as Fix_8_3 before applying a UFix_8_4 gain
      | which gives Fix_16_7.  The Fix_16_7 value is cast
      | to a Fix_4_0 with saturation and round-to-even.
  g : Inport
  in8 : Inport  Port=2
  Mult : xbsIndex_r4/Mult  precision=Full  arith_type="Signed  (2's comp)"  n_bits=16  bin_pt=14  quantization=Truncate  overflow=Wrap  en=off  latency=3  dbl_ovrd=off  use_behavioral_HDL=off  opt=Speed  use_embedded=on  optimum_pipeline=off  pipeline=on  use_rpm=on  placement_style=Triangular  block_type=mult
  Reinterpret1 : xbsIndex_r4/Reinterpret  force_arith_type=off  arith_type=Unsigned  force_bin_pt=on  bin_pt=3  block_type=reinterpret
  convert : Subsystem  AncestorBlock=casper_library_misc/convert  mask(convert){bin_pt_in=7, n_bits_out=4, bin_pt_out=0, quantization=Round  (unbiased: Even Values), overflow=Saturate, latency=4}
      mask prompts: bin_pt_in "Binary Point In"; n_bits_out "Bit Width Out"; bin_pt_out "Binary Point Out"
      mask help:
      | Convert input value to the specified bit width and precision.
      |
      | Does not support upscaling number of fractional bits (eg 8_5 to 8_4 is ok, but not 8_4 to 8_5).
      mask init: same as sma_corr/cgain_0/convert
      settings: AttributesFormatString=|
      | N_7 ==> 4_0
      | round even, saturate
      | latency = 4
      mask Help:
      | <pre>
      | Description::
      | 
      | Outputs converted version of the input.
      | 
      | Mask Parameters::
      | 
      | Binary Point In (bin_pt_in): Specifies the binary point location in the input.
      | 
      | Bit Width Out (n_bits_out): Specifies the width of the output.
      | 
      | Binary Point Out (bin_pt_out): Specifies the binary point location in the output.
      | 
      | Quantization (quantization): Specifies quantization handling.
      | 
      | Overflow (overflow): Specifies overflow handling.
      | 
      | Latency (latency): Specifies the latency of the underlying adder block, which is the overall
      | latency of this block as well.
      | </pre>
      mask TypeOptions quantization: Truncate | Round  (unbiased: +/- Inf) | Round  (unbiased: Even Values) | Round  (unbiased: Zero) | Round  (unbiased: Odd Values) | Round  (biased: Up) | Round  (biased: Down)
      mask TypeOptions overflow: Wrap | Saturate | Error
  out4 : Outport
  wires:
  g/1 -> Mult/1
  in8/1 -> Reinterpret1/1
  Mult/1 -> convert/1(in)
  Reinterpret1/1 -> Mult/2
  convert/1(out) -> out4/1

== system sma_corr/beng/sum2/sum_Ycd/gain_and_quantize1 ==
  note: w,x,y,z are Fix_8_0, but the MSb is redundant
      | (they are really only Fix_7_0).  We want to keep
      | the top 4 of those seven bits, so we reinterpret
      | them as Fix_8_3 before applying a UFix_8_4 gain
      | which gives Fix_16_7.  The Fix_16_7 value is cast
      | to a Fix_4_0 with saturation and round-to-even.
  g : Inport
  in8 : Inport  Port=2
  Mult : xbsIndex_r4/Mult  precision=Full  arith_type="Signed  (2's comp)"  n_bits=16  bin_pt=14  quantization=Truncate  overflow=Wrap  en=off  latency=3  dbl_ovrd=off  use_behavioral_HDL=off  opt=Speed  use_embedded=on  optimum_pipeline=off  pipeline=on  use_rpm=on  placement_style=Triangular  block_type=mult
  Reinterpret1 : xbsIndex_r4/Reinterpret  force_arith_type=off  arith_type=Unsigned  force_bin_pt=on  bin_pt=3  block_type=reinterpret
  convert : Subsystem  AncestorBlock=casper_library_misc/convert  mask(convert){bin_pt_in=7, n_bits_out=4, bin_pt_out=0, quantization=Round  (unbiased: Even Values), overflow=Saturate, latency=4}
      mask prompts: bin_pt_in "Binary Point In"; n_bits_out "Bit Width Out"; bin_pt_out "Binary Point Out"
      mask help:
      | Convert input value to the specified bit width and precision.
      |
      | Does not support upscaling number of fractional bits (eg 8_5 to 8_4 is ok, but not 8_4 to 8_5).
      mask init: same as sma_corr/cgain_0/convert
      settings: AttributesFormatString=|
      | N_7 ==> 4_0
      | round even, saturate
      | latency = 4
      mask Help:
      | <pre>
      | Description::
      | 
      | Outputs converted version of the input.
      | 
      | Mask Parameters::
      | 
      | Binary Point In (bin_pt_in): Specifies the binary point location in the input.
      | 
      | Bit Width Out (n_bits_out): Specifies the width of the output.
      | 
      | Binary Point Out (bin_pt_out): Specifies the binary point location in the output.
      | 
      | Quantization (quantization): Specifies quantization handling.
      | 
      | Overflow (overflow): Specifies overflow handling.
      | 
      | Latency (latency): Specifies the latency of the underlying adder block, which is the overall
      | latency of this block as well.
      | </pre>
      mask TypeOptions quantization: Truncate | Round  (unbiased: +/- Inf) | Round  (unbiased: Even Values) | Round  (unbiased: Zero) | Round  (unbiased: Odd Values) | Round  (biased: Up) | Round  (biased: Down)
      mask TypeOptions overflow: Wrap | Saturate | Error
  out4 : Outport
  wires:
  convert/1(out) -> out4/1
  Reinterpret1/1 -> Mult/2
  Mult/1 -> convert/1(in)
  in8/1 -> Reinterpret1/1
  g/1 -> Mult/1

== system sma_corr/beng/sum2/sum_Ycd/gain_and_quantize2 ==
  note: w,x,y,z are Fix_8_0, but the MSb is redundant
      | (they are really only Fix_7_0).  We want to keep
      | the top 4 of those seven bits, so we reinterpret
      | them as Fix_8_3 before applying a UFix_8_4 gain
      | which gives Fix_16_7.  The Fix_16_7 value is cast
      | to a Fix_4_0 with saturation and round-to-even.
  g : Inport
  in8 : Inport  Port=2
  Mult : xbsIndex_r4/Mult  precision=Full  arith_type="Signed  (2's comp)"  n_bits=16  bin_pt=14  quantization=Truncate  overflow=Wrap  en=off  latency=3  dbl_ovrd=off  use_behavioral_HDL=off  opt=Speed  use_embedded=on  optimum_pipeline=off  pipeline=on  use_rpm=on  placement_style=Triangular  block_type=mult
  Reinterpret1 : xbsIndex_r4/Reinterpret  force_arith_type=off  arith_type=Unsigned  force_bin_pt=on  bin_pt=3  block_type=reinterpret
  convert : Subsystem  AncestorBlock=casper_library_misc/convert  mask(convert){bin_pt_in=7, n_bits_out=4, bin_pt_out=0, quantization=Round  (unbiased: Even Values), overflow=Saturate, latency=4}
      mask prompts: bin_pt_in "Binary Point In"; n_bits_out "Bit Width Out"; bin_pt_out "Binary Point Out"
      mask help:
      | Convert input value to the specified bit width and precision.
      |
      | Does not support upscaling number of fractional bits (eg 8_5 to 8_4 is ok, but not 8_4 to 8_5).
      mask init: same as sma_corr/cgain_0/convert
      settings: AttributesFormatString=|
      | N_7 ==> 4_0
      | round even, saturate
      | latency = 4
      mask Help:
      | <pre>
      | Description::
      | 
      | Outputs converted version of the input.
      | 
      | Mask Parameters::
      | 
      | Binary Point In (bin_pt_in): Specifies the binary point location in the input.
      | 
      | Bit Width Out (n_bits_out): Specifies the width of the output.
      | 
      | Binary Point Out (bin_pt_out): Specifies the binary point location in the output.
      | 
      | Quantization (quantization): Specifies quantization handling.
      | 
      | Overflow (overflow): Specifies overflow handling.
      | 
      | Latency (latency): Specifies the latency of the underlying adder block, which is the overall
      | latency of this block as well.
      | </pre>
      mask TypeOptions quantization: Truncate | Round  (unbiased: +/- Inf) | Round  (unbiased: Even Values) | Round  (unbiased: Zero) | Round  (unbiased: Odd Values) | Round  (biased: Up) | Round  (biased: Down)
      mask TypeOptions overflow: Wrap | Saturate | Error
  out4 : Outport
  wires:
  g/1 -> Mult/1
  in8/1 -> Reinterpret1/1
  Mult/1 -> convert/1(in)
  Reinterpret1/1 -> Mult/2
  convert/1(out) -> out4/1

== system sma_corr/beng/sum2/sum_Ycd/gain_and_quantize3 ==
  note: w,x,y,z are Fix_8_0, but the MSb is redundant
      | (they are really only Fix_7_0).  We want to keep
      | the top 4 of those seven bits, so we reinterpret
      | them as Fix_8_3 before applying a UFix_8_4 gain
      | which gives Fix_16_7.  The Fix_16_7 value is cast
      | to a Fix_4_0 with saturation and round-to-even.
  g : Inport
  in8 : Inport  Port=2
  Mult : xbsIndex_r4/Mult  precision=Full  arith_type="Signed  (2's comp)"  n_bits=16  bin_pt=14  quantization=Truncate  overflow=Wrap  en=off  latency=3  dbl_ovrd=off  use_behavioral_HDL=off  opt=Speed  use_embedded=on  optimum_pipeline=off  pipeline=on  use_rpm=on  placement_style=Triangular  block_type=mult
  Reinterpret1 : xbsIndex_r4/Reinterpret  force_arith_type=off  arith_type=Unsigned  force_bin_pt=on  bin_pt=3  block_type=reinterpret
  convert : Subsystem  AncestorBlock=casper_library_misc/convert  mask(convert){bin_pt_in=7, n_bits_out=4, bin_pt_out=0, quantization=Round  (unbiased: Even Values), overflow=Saturate, latency=4}
      mask prompts: bin_pt_in "Binary Point In"; n_bits_out "Bit Width Out"; bin_pt_out "Binary Point Out"
      mask help:
      | Convert input value to the specified bit width and precision.
      |
      | Does not support upscaling number of fractional bits (eg 8_5 to 8_4 is ok, but not 8_4 to 8_5).
      mask init: same as sma_corr/cgain_0/convert
      settings: AttributesFormatString=|
      | N_7 ==> 4_0
      | round even, saturate
      | latency = 4
      mask Help:
      | <pre>
      | Description::
      | 
      | Outputs converted version of the input.
      | 
      | Mask Parameters::
      | 
      | Binary Point In (bin_pt_in): Specifies the binary point location in the input.
      | 
      | Bit Width Out (n_bits_out): Specifies the width of the output.
      | 
      | Binary Point Out (bin_pt_out): Specifies the binary point location in the output.
      | 
      | Quantization (quantization): Specifies quantization handling.
      | 
      | Overflow (overflow): Specifies overflow handling.
      | 
      | Latency (latency): Specifies the latency of the underlying adder block, which is the overall
      | latency of this block as well.
      | </pre>
      mask TypeOptions quantization: Truncate | Round  (unbiased: +/- Inf) | Round  (unbiased: Even Values) | Round  (unbiased: Zero) | Round  (unbiased: Odd Values) | Round  (biased: Up) | Round  (biased: Down)
      mask TypeOptions overflow: Wrap | Saturate | Error
  out4 : Outport
  wires:
  convert/1(out) -> out4/1
  Reinterpret1/1 -> Mult/2
  Mult/1 -> convert/1(in)
  in8/1 -> Reinterpret1/1
  g/1 -> Mult/1

== system sma_corr/beng/sum2/sum_Ycd/simd_vacc ==
  note: Note that this bit basher takes the upper 8 bits of every 12, as opposed to the
      | lower 8, which is the case in .../sum[01]/sum_???/simd_vac (sideband0 path).
      | Here the upper 8 are needed, since we accumulate 8-bit data over 8 cycles -> grows to 12-bit;
      | there the lower 8 are needed, since it accumulates 4-bit data over 8 cycles -> grows to 8-bit.
  note: First "acc_init" pulse after sync does not indicate valid.
      | Hold off "valid" output until after the first falling edge of
      | acc_init that is after the sync pulse.
  note: Accummulate 8 vectors of length 128 samples.
      | Do four such accumulations in parallel.
  sync : Inport
  in : Inport  Port=2
  disable : Inport  Port=3
  BitBasher2 : xbsIndex_r4/BitBasher  bitexpr=|  display_expr=off  sr_1=1  arith_type1="Signed  (2's comp)"  bin_pt1=0  sr_2=2  arith_type2="Signed  (2's comp)"  bin_pt2=0  sr_3=3  arith_type3="Signed  (2's comp)"  bin_pt3=0  sr_4=4  arith_type4="Signed  (2's comp)"  bin_pt4=0  dbl_ovrd=off  block_type=bitbasher
      | <!DOCTYPE HTML PUBLIC "-//W3C//DTD HTML 4.0//EN" "http://www.w3.org/TR/REC-html40/strict.dtd">
      | <html><head><meta name="qrichtext" content="1" /><style type="text/css">
      | p, li { white-space: pre-wrap; }
      | </style></head><body style=" font-family:'Ubuntu'; font-size:11pt; font-weight:400; font-style:normal;">
      | <p style=" margin-top:12px; margin-bottom:12px; margin-left:0px; margin-right:0px; -qt-block-indent:0; text-indent:0px;"><span style=" font-family:'MS Shell Dlg'; font-size:8pt;">w={p[47:40]}<br />x={p[35:28]}<br />y={p[23:16]}<br />z={p[11:04]}<br /></span></p></body></html>
  Constant1 : xbsIndex_r4/Constant  const=1  gui_display_data_type=Boolean  arith_type=Boolean  n_bits=16  bin_pt=14  preci_type=Single  exp_width=8  frac_width=24  explicit_period=on  period=1  equ=P=C  opselect=C  inp2=PCIN>>17  opr=+  inp1=P  carry=CIN  dbl_ovrd=off  block_type=constant
  Delay{1..4} (4 alike) : xbsIndex_r4/Delay  rst=off  infoeditControl=Selection of Reset will increase slice count due to use of real FFs and instead of SRLs  en=off  latency=1  dbl_ovrd=off  reg_retiming=off  block_type=delay
  Register1 : xbsIndex_r4/Register  init=0  rst=on  en=on  dbl_ovrd=off  block_type=register
  acc4par : Subsystem
  armed_trigger : casper_library_misc/armed_trigger
  controller : Subsystem
  delay_bram : Subsystem  AncestorBlock=casper_library_delays/delay_bram  mask(delay_bram){DelayLen=128-4, bram_latency=3, use_dsp48=off, async=off}
      mask prompts: DelayLen "Delay By:"; bram_latency "BRAM Latency"; use_dsp48 "Use DSP48"; async "asynchronous operation"
      mask help: A delay block that uses BRAM for its storage.
      mask init:
      | delay_bram_init(gcb, ...
      |     'DelayLen', DelayLen, ...
      |     'bram_latency', bram_latency, ...
      |     'use_dsp48', use_dsp48, ...
      |     'async', async);
      mask Help: eval('xlWeb(''http://casper.berkeley.edu/wiki/Delay_bram'')')
  edge_detect : Subsystem  AncestorBlock=casper_library_misc/edge_detect  mask(edge_detect){edge=Falling, polarity=Active High, x_in=[0 0.08 0.08 0.16 0.16 0.24 0.24 0.32 0.32 0.4], y_in=[-1 -1  1  1  1  1  1  1  1  1], x_out=[0.6 0.68 0.68 0.76 0.76 0.84 0.84 0.92 0.92 1], y_out=[-1 -1  1  1 -1 -1 -1 -1 -1 -1]}
      mask prompts: edge "Edge Type"; polarity "Output Polarity"; x_in "Input X Positions"; y_in "Input Y Positions"; x_out "Output X Positions"; y_out "Output Y Positions"
      mask help: Output is active for one-cycle after each edge of the specified type (i.e. rising, falling or both).
      mask init: same as sma_corr/scope/edge_detect
      settings: AttributesFormatString=|
      | falling edges
      | active high
      mask Help: Output is active for one-cycle after each edge of the specified type (i.e. rising, falling, or both).  Latency is zero.
      mask TypeOptions edge: Rising | Falling | Both
      mask TypeOptions polarity: Active High | Active Low
      mask Display:
      | plot(x_in_num,0.5+y_in_num*0.2);
      | plot(x_out_num,0.5+y_out_num*0.2);
  valid_holdoff : xbsIndex_r4/Logical  logical_function=AND  inputs=2  en=off  latency=1  precision=Full  arith_type=Unsigned  n_bits=16  bin_pt=0  align_bp=on  dbl_ovrd=off  block_type=logical
  sync_out : Outport
  valid : Outport  Port=2
  w : Outport  Port=3
  x : Outport  Port=4
  y : Outport  Port=5
  z : Outport  Port=6
  wires:
  in/1 -> Delay3/1
  delay_bram/1(dout) -> Delay2/1
  acc4par/1(out48) -> Delay1/1
  Delay1/1 -> delay_bram/1(din)
  Delay2/1 -> acc4par/4(acc48), Delay4/1
  Delay4/1 -> BitBasher2/1
  edge_detect/1(out) -> Register1/3
  Constant1/1 -> Register1/1
  Register1/1 -> armed_trigger/1, valid_holdoff/2
  controller/2(acc_init) -> edge_detect/1(in), acc4par/3(acc_init), valid_holdoff/1, armed_trigger/2
  sync/1 -> Register1/2, controller/2(sync)
  disable/1 -> controller/1(disable)
  controller/1(zero_op) -> acc4par/2(zero_input)
  Delay3/1 -> acc4par/1(in32)
  valid_holdoff/1 -> valid/1
  armed_trigger/1 -> sync_out/1
  BitBasher2/1 -> w/1
  BitBasher2/4 -> z/1
  BitBasher2/2 -> x/1
  BitBasher2/3 -> y/1
